simulink model "large_model"  (Simulink 8.0)
settings: Solver=ode45  StartTime=0.0  StopTime=10.0  RelTol=1e-3
(part 10 of 21)

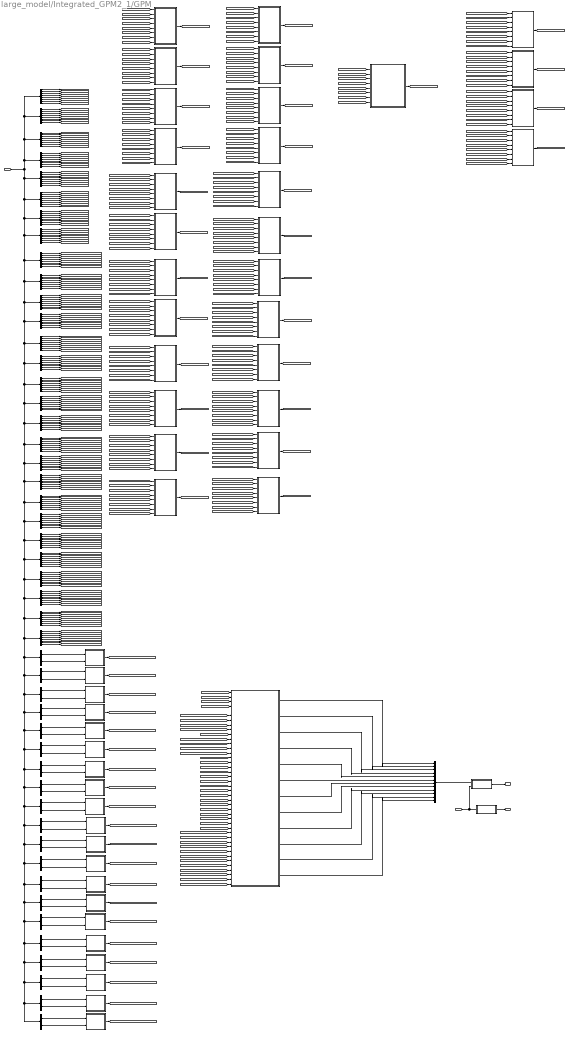
== system large_model/Integrated_GPM2_1/GPM ==
  note: Este componente foi colocado devido a uma limitação do EFTG. Na versão 1.2.1,
      | não é permitido fazer uma conexão direta entre a entrada e a saída.
  From_ES : Inport
  PWR_In : Inport  Port=2
  CAB1_FCC2 : Subsystem
  GPM Hardware : Subsystem
  Outputs : BusCreator  Inputs=12
  PWR : Subsystem
  SCP_REU_ROB_LVDT : Subsystem
  SCP_ail_REU_LIB_LVDT : Subsystem
  SCP_ail_REU_LIB_mon : Subsystem
  SCP_ail_REU_LOB_LVDT : Subsystem
  SCP_ail_REU_LOB_mon : Subsystem
  SCP_ail_REU_RIB_LVDT : Subsystem
  SCP_ail_REU_RIB_mon : Subsystem
  SCP_ail_REU_ROB_mon : Subsystem
  SCP_elev_REU_LIB_LVDT : Subsystem
  SCP_elev_REU_LIB_mon_dat : Subsystem
  SCP_elev_REU_LOB_LVDT : Subsystem
  SCP_elev_REU_LOB_mon : Subsystem
  SCP_elev_REU_RIB_LVDT_dat : Subsystem
  SCP_elev_REU_RIB_mon : Subsystem
  SCP_elev_REU_ROB_LVDT : Subsystem
  SCP_elev_REU_ROB_mon : Subsystem
  SCP_rud_REU1_LVDT : Subsystem
  SCP_rud_REU1_MON : Subsystem
  SCP_rud_REU2_LVDT : Subsystem
  SCP_rud_REU2_MON : Subsystem
  Self_checking_pair_ADS1A : Subsystem
  Self_checking_pair_ADS1B : Subsystem
  Self_checking_pair_ADS2A : Subsystem
  Self_checking_pair_ADS2B : Subsystem
  Self_checking_pair_ADS3A : Subsystem
  Self_checking_pair_ADS3B : Subsystem
  Self_checking_pair_ADS4A : Subsystem
  Self_checking_pair_ADS4B : Subsystem
  Self_checking_pair_PedalYaw1 : Subsystem
  Self_checking_pair_PedalYaw2 : Subsystem
  Self_checking_pair_PedalYaw3 : Subsystem
  Self_checking_pair_PedalYaw4 : Subsystem
  Self_checking_pair_SSC1_pitch1 : Subsystem
  Self_checking_pair_SSC1_pitch2 : Subsystem
  Self_checking_pair_SSC1_pitch3 : Subsystem
  Self_checking_pair_SSC1_pitch4 : Subsystem
  Self_checking_pair_SSC1_roll1 : Subsystem
  Self_checking_pair_SSC1_roll2 : Subsystem
  Self_checking_pair_SSC1_roll3 : Subsystem
  Self_checking_pair_SSC1_roll4 : Subsystem
  Self_checking_pair_SSC2_pitch1 : Subsystem
  Self_checking_pair_SSC2_pitch2 : Subsystem
  Self_checking_pair_SSC2_pitch3 : Subsystem
  Self_checking_pair_SSC2_pitch4 : Subsystem
  Self_checking_pair_SSC2_roll1 : Subsystem
  Self_checking_pair_SSC2_roll2 : Subsystem
  Self_checking_pair_SSC2_roll3 : Subsystem
  Self_checking_pair_SSC2_roll4 : Subsystem
  Voter_ADS : Subsystem
  ads_1A : BusSelector  Description=|  OutputSignals=switch1A.cab2_gpm_IO_chB.ads_1A,switch1A.cab1_gpm_IO_chB.ads_1A,switch1A.switch2A_to_switch.cab3_gpm_IO_chA.ads_1A,switch1A.switch2A_to_switch.cab4_gpm_IO_chA.ads_1A,switch1B.cab2_gpm_IO_chB.ads_1A,switch1B.cab1_gpm_IO_chB.ads_1A,switch1B.switch2B_to_switch.cab4_gpm_IO_chB.ads_1A,switch1B.switch2B_to_switch.cab3_gpm_IO_chB.ads_1A
      | When connected to a line which contains bus signals, this bus selector will select as its outputs all the signals which contain this bus selector name.
      | For example, if you name this bus selector as "sidestick_1_pitch_1", all signals containing this string will be selected as its outputs.
  ads_1B : BusSelector  Description=|  OutputSignals=switch1A.cab2_gpm_IO_chB.ads_1B,switch1A.cab1_gpm_IO_chB.ads_1B,switch1A.switch2A_to_switch.cab3_gpm_IO_chA.ads_1B,switch1A.switch2A_to_switch.cab4_gpm_IO_chA.ads_1B,switch1B.cab2_gpm_IO_chB.ads_1B,switch1B.cab1_gpm_IO_chB.ads_1B,switch1B.switch2B_to_switch.cab4_gpm_IO_chB.ads_1B,switch1B.switch2B_to_switch.cab3_gpm_IO_chB.ads_1B
      | When connected to a line which contains bus signals, this bus selector will select as its outputs all the signals which contain this bus selector name.
      | For example, if you name this bus selector as "sidestick_1_pitch_1", all signals containing this string will be selected as its outputs.
  ads_2A : BusSelector  Description=|  OutputSignals=switch1A.cab2_gpm_IO_chB.ads_2A,switch1A.cab1_gpm_IO_chB.ads_2A,switch1A.switch2A_to_switch.cab3_gpm_IO_chA.ads_2A,switch1A.switch2A_to_switch.cab4_gpm_IO_chA.ads_2A,switch1B.cab2_gpm_IO_chB.ads_2A,switch1B.cab1_gpm_IO_chB.ads_2A,switch1B.switch2B_to_switch.cab4_gpm_IO_chB.ads_2A,switch1B.switch2B_to_switch.cab3_gpm_IO_chB.ads_2A
      | When connected to a line which contains bus signals, this bus selector will select as its outputs all the signals which contain this bus selector name.
      | For example, if you name this bus selector as "sidestick_1_pitch_1", all signals containing this string will be selected as its outputs.
  ads_2B : BusSelector  Description=|  OutputSignals=switch1A.cab2_gpm_IO_chB.ads_2B,switch1A.cab1_gpm_IO_chB.ads_2B,switch1A.switch2A_to_switch.cab3_gpm_IO_chA.ads_2B,switch1A.switch2A_to_switch.cab4_gpm_IO_chA.ads_2B,switch1B.cab2_gpm_IO_chB.ads_2B,switch1B.cab1_gpm_IO_chB.ads_2B,switch1B.switch2B_to_switch.cab4_gpm_IO_chB.ads_2B,switch1B.switch2B_to_switch.cab3_gpm_IO_chB.ads_2B
      | When connected to a line which contains bus signals, this bus selector will select as its outputs all the signals which contain this bus selector name.
      | For example, if you name this bus selector as "sidestick_1_pitch_1", all signals containing this string will be selected as its outputs.
  ads_3A : BusSelector  Description=|  OutputSignals=switch1A.cab2_gpm_IO_chB.ads_3A,switch1A.cab1_gpm_IO_chB.ads_3A,switch1A.switch2A_to_switch.cab3_gpm_IO_chA.ads_3A,switch1A.switch2A_to_switch.cab4_gpm_IO_chA.ads_3A,switch1B.cab2_gpm_IO_chB.ads_3A,switch1B.cab1_gpm_IO_chB.ads_3A,switch1B.switch2B_to_switch.cab4_gpm_IO_chB.ads_3A,switch1B.switch2B_to_switch.cab3_gpm_IO_chB.ads_3A
      | When connected to a line which contains bus signals, this bus selector will select as its outputs all the signals which contain this bus selector name.
      | For example, if you name this bus selector as "sidestick_1_pitch_1", all signals containing this string will be selected as its outputs.
  ads_3B : BusSelector  Description=|  OutputSignals=switch1A.cab2_gpm_IO_chB.ads_3B,switch1A.cab1_gpm_IO_chB.ads_3B,switch1A.switch2A_to_switch.cab3_gpm_IO_chA.ads_3B,switch1A.switch2A_to_switch.cab4_gpm_IO_chA.ads_3B,switch1B.cab2_gpm_IO_chB.ads_3B,switch1B.cab1_gpm_IO_chB.ads_3B,switch1B.switch2B_to_switch.cab4_gpm_IO_chB.ads_3B,switch1B.switch2B_to_switch.cab3_gpm_IO_chB.ads_3B
      | When connected to a line which contains bus signals, this bus selector will select as its outputs all the signals which contain this bus selector name.
      | For example, if you name this bus selector as "sidestick_1_pitch_1", all signals containing this string will be selected as its outputs.
  ads_4A : BusSelector  Description=|  OutputSignals=switch1A.cab2_gpm_IO_chB.ads_4A,switch1A.cab1_gpm_IO_chB.ads_4A,switch1A.switch2A_to_switch.cab3_gpm_IO_chA.ads_4A,switch1A.switch2A_to_switch.cab4_gpm_IO_chA.ads_4A,switch1B.cab2_gpm_IO_chB.ads_4A,switch1B.cab1_gpm_IO_chB.ads_4A,switch1B.switch2B_to_switch.cab4_gpm_IO_chB.ads_4A,switch1B.switch2B_to_switch.cab3_gpm_IO_chB.ads_4A
      | When connected to a line which contains bus signals, this bus selector will select as its outputs all the signals which contain this bus selector name.
      | For example, if you name this bus selector as "sidestick_1_pitch_1", all signals containing this string will be selected as its outputs.
  ads_4B : BusSelector  Description=|  OutputSignals=switch1A.cab2_gpm_IO_chB.ads_4B,switch1A.cab1_gpm_IO_chB.ads_4B,switch1A.switch2A_to_switch.cab3_gpm_IO_chA.ads_4B,switch1A.switch2A_to_switch.cab4_gpm_IO_chA.ads_4B,switch1B.cab2_gpm_IO_chB.ads_4B,switch1B.cab1_gpm_IO_chB.ads_4B,switch1B.switch2B_to_switch.cab4_gpm_IO_chB.ads_4B,switch1B.switch2B_to_switch.cab3_gpm_IO_chB.ads_4B
      | When connected to a line which contains bus signals, this bus selector will select as its outputs all the signals which contain this bus selector name.
      | For example, if you name this bus selector as "sidestick_1_pitch_1", all signals containing this string will be selected as its outputs.
  ail_REU_LIB_LVDT_dat : BusSelector  OutputSignals=switch1A.switch2A_to_switch.REU_to_Switch2A.ail_REU_LIB_LVDT_dat,switch1B.switch2B_to_switch.REU_to_Switch2B.ail_REU_LIB_LVDT_dat
  ail_REU_LIB_mon_dat : BusSelector  OutputSignals=switch1A.switch2A_to_switch.REU_to_Switch2A.ail_REU_LIB_mon_dat,switch1B.switch2B_to_switch.REU_to_Switch2B.ail_REU_LIB_mon_dat
  ail_REU_LOB_LVDT_dat : BusSelector  OutputSignals=switch1A.REU_to_Switch1A.ail_REU_LOB_LVDT_dat,switch1B.REU_to_Switch1B.ail_REU_LOB_LVDT_dat
  ail_REU_LOB_mon_dat : BusSelector  OutputSignals=switch1A.REU_to_Switch1A.ail_REU_LOB_mon_dat,switch1B.REU_to_Switch1B.ail_REU_LOB_mon_dat
  ail_REU_RIB_LVDT_dat : BusSelector  OutputSignals=switch1A.switch2A_to_switch.REU_to_Switch2A.ail_REU_RIB_LVDT_dat,switch1B.switch2B_to_switch.REU_to_Switch2B.ail_REU_RIB_LVDT_dat
  ail_REU_RIB_mon_dat : BusSelector  OutputSignals=switch1A.switch2A_to_switch.REU_to_Switch2A.ail_REU_RIB_mon_dat,switch1B.switch2B_to_switch.REU_to_Switch2B.ail_REU_RIB_mon_dat
  ail_REU_ROB_LVDT_dat : BusSelector  OutputSignals=switch1A.REU_to_Switch1A.ail_REU_ROB_LVDT_dat,switch1B.REU_to_Switch1B.ail_REU_ROB_LVDT_dat
  ail_REU_ROB_mon_dat : BusSelector  OutputSignals=switch1A.REU_to_Switch1A.ail_REU_ROB_mon_dat,switch1B.REU_to_Switch1B.ail_REU_ROB_mon_dat
  elev_REU_LIB_LVDT_dat : BusSelector  OutputSignals=switch1A.switch2A_to_switch.REU_to_Switch2A.elev_REU_LIB_LVDT_dat,switch1B.switch2B_to_switch.REU_to_Switch2B.elev_REU_LIB_LVDT_dat
  elev_REU_LIB_mon_dat : BusSelector  OutputSignals=switch1A.switch2A_to_switch.REU_to_Switch2A.elev_REU_LIB_mon_dat,switch1B.switch2B_to_switch.REU_to_Switch2B.elev_REU_LIB_mon_dat
  elev_REU_LOB_LVDT_dat : BusSelector  OutputSignals=switch1A.REU_to_Switch1A.elev_REU_LOB_LVDT_dat,switch1B.REU_to_Switch1B.elev_REU_LOB_LVDT_dat
  elev_REU_LOB_mon_dat : BusSelector  OutputSignals=switch1A.REU_to_Switch1A.elev_REU_LOB_mon_dat,switch1B.REU_to_Switch1B.elev_REU_LOB_mon_dat
  elev_REU_RIB_LVDT_dat : BusSelector  OutputSignals=switch1A.switch2A_to_switch.REU_to_Switch2A.elev_REU_RIB_LVDT_dat,switch1B.switch2B_to_switch.REU_to_Switch2B.elev_REU_RIB_LVDT_dat
  elev_REU_RIB_mon_dat : BusSelector  OutputSignals=switch1A.switch2A_to_switch.REU_to_Switch2A.elev_REU_RIB_mon_dat,switch1B.switch2B_to_switch.REU_to_Switch2B.elev_REU_RIB_mon_dat
  elev_REU_ROB_LVDT_dat : BusSelector  OutputSignals=switch1A.REU_to_Switch1A.elev_REU_ROB_LVDT_dat,switch1B.REU_to_Switch1B.elev_REU_ROB_LVDT_dat
  elev_REU_ROB_mon_dat : BusSelector  OutputSignals=switch1A.REU_to_Switch1A.elev_REU_ROB_mon_dat,switch1B.REU_to_Switch1B.elev_REU_ROB_mon_dat
  pedal_yaw_1 : BusSelector  Description=|  OutputSignals=switch1A.cab2_gpm_IO_chB.rdc_4002_1.pedal_yaw_1,switch1A.cab1_gpm_IO_chB.rdc_4002_1.pedal_yaw_1,switch1A.switch2A_to_switch.cab3_gpm_IO_chA.rdc_4002_1.pedal_yaw_1,switch1A.switch2A_to_switch.cab4_gpm_IO_chA.rdc_4002_1.pedal_yaw_1,switch1B.cab2_gpm_IO_chB.rdc_4002_1.pedal_yaw_1,switch1B.cab1_gpm_IO_chB.rdc_4002_1.pedal_yaw_1,switch1B.switch2B_to_switch.cab4_gpm_IO_chB.rdc_4002_1.pedal_yaw_1,switch1B.switch2B_to_switch.cab3_gpm_IO_chB.rdc_4002_1.pedal_yaw_1
      | When connected to a line which contains bus signals, this bus selector will select as its outputs all the signals which contain this bus selector name.
      | For example, if you name this bus selector as "sidestick_1_pitch_1", all signals containing this string will be selected as its outputs.
  pedal_yaw_2 : BusSelector  Description=|  OutputSignals=switch1A.cab2_gpm_IO_chB.rdc_4002_2.pedal_yaw_2,switch1A.cab1_gpm_IO_chB.rdc_4002_2.pedal_yaw_2,switch1A.switch2A_to_switch.cab3_gpm_IO_chA.rdc_4002_2.pedal_yaw_2,switch1A.switch2A_to_switch.cab4_gpm_IO_chA.rdc_4002_2.pedal_yaw_2,switch1B.cab2_gpm_IO_chB.rdc_4002_2.pedal_yaw_2,switch1B.cab1_gpm_IO_chB.rdc_4002_2.pedal_yaw_2,switch1B.switch2B_to_switch.cab4_gpm_IO_chB.rdc_4002_2.pedal_yaw_2,switch1B.switch2B_to_switch.cab3_gpm_IO_chB.rdc_4002_2.pedal_yaw_2
      | When connected to a line which contains bus signals, this bus selector will select as its outputs all the signals which contain this bus selector name.
      | For example, if you name this bus selector as "sidestick_1_pitch_1", all signals containing this string will be selected as its outputs.
  pedal_yaw_3 : BusSelector  Description=|  OutputSignals=switch1A.cab2_gpm_IO_chB.rdc_4002_3.pedal_yaw_3,switch1A.cab1_gpm_IO_chB.rdc_4002_3.pedal_yaw_3,switch1A.switch2A_to_switch.cab3_gpm_IO_chA.rdc_4002_3.pedal_yaw_3,switch1A.switch2A_to_switch.cab4_gpm_IO_chA.rdc_4002_3.pedal_yaw_3,switch1B.cab2_gpm_IO_chB.rdc_4002_3.pedal_yaw_3,switch1B.cab1_gpm_IO_chB.rdc_4002_3.pedal_yaw_3,switch1B.switch2B_to_switch.cab4_gpm_IO_chB.rdc_4002_3.pedal_yaw_3,switch1B.switch2B_to_switch.cab3_gpm_IO_chB.rdc_4002_3.pedal_yaw_3
      | When connected to a line which contains bus signals, this bus selector will select as its outputs all the signals which contain this bus selector name.
      | For example, if you name this bus selector as "sidestick_1_pitch_1", all signals containing this string will be selected as its outputs.
  pedal_yaw_4 : BusSelector  Description=|  OutputSignals=switch1A.cab2_gpm_IO_chB.rdc_4002_4.pedal_yaw_4,switch1A.cab1_gpm_IO_chB.rdc_4002_4.pedal_yaw_4,switch1A.switch2A_to_switch.cab3_gpm_IO_chA.rdc_4002_4.pedal_yaw_4,switch1A.switch2A_to_switch.cab4_gpm_IO_chA.rdc_4002_4.pedal_yaw_4,switch1B.cab2_gpm_IO_chB.rdc_4002_4.pedal_yaw_4,switch1B.cab1_gpm_IO_chB.rdc_4002_4.pedal_yaw_4,switch1B.switch2B_to_switch.cab4_gpm_IO_chB.rdc_4002_4.pedal_yaw_4,switch1B.switch2B_to_switch.cab3_gpm_IO_chB.rdc_4002_4.pedal_yaw_4
      | When connected to a line which contains bus signals, this bus selector will select as its outputs all the signals which contain this bus selector name.
      | For example, if you name this bus selector as "sidestick_1_pitch_1", all signals containing this string will be selected as its outputs.
  rud_REU1_LVDT_dat : BusSelector  OutputSignals=switch1A.switch2A_to_switch.REU_to_Switch2A.rud_REU1_LVDT_dat,switch1B.switch2B_to_switch.REU_to_Switch2B.rud_REU1_LVDT_dat
  rud_REU1_MON_dat : BusSelector  OutputSignals=switch1A.switch2A_to_switch.REU_to_Switch2A.rud_REU1_MON_dat,switch1B.switch2B_to_switch.REU_to_Switch2B.rud_REU1_MON_dat
  rud_REU2_LVDT_dat : BusSelector  OutputSignals=switch1A.REU_to_Switch1A.rud_REU2_LVDT_dat,switch1B.REU_to_Switch1B.rud_REU2_LVDT_dat
  rud_REU2_MON_dat : BusSelector  OutputSignals=switch1A.REU_to_Switch1A.rud_REU2_MON_dat,switch1B.REU_to_Switch1B.rud_REU2_MON_dat
  sidestick1_pitch_1 : BusSelector  OutputSignals=switch1A.cab2_gpm_IO_chB.rdc_4002_1.sidesticks.sidestick1_pitch_1,switch1A.cab1_gpm_IO_chB.rdc_4002_1.sidesticks.sidestick1_pitch_1,switch1A.switch2A_to_switch.cab3_gpm_IO_chA.rdc_4002_1.sidesticks.sidestick1_pitch_1,switch1A.switch2A_to_switch.cab4_gpm_IO_chA.rdc_4002_1.sidesticks.sidestick1_pitch_1,switch1B.cab2_gpm_IO_chB.rdc_4002_1.sidesticks.sidestick1_pitch_1,switch1B.cab1_gpm_IO_chB.rdc_4002_1.sidesticks.sidestick1_pitch_1,switch1B.switch2B_to_switch.cab4_gpm_IO_chB.rdc_4002_1.sidesticks.sidestick1_pitch_1,switch1B.switch2B_to_switch.cab3_gpm_IO_chB.rdc_4002_1.sidesticks.sidestick1_pitch_1
  sidestick1_pitch_2 : BusSelector  OutputSignals=switch1A.cab2_gpm_IO_chB.rdc_4002_2.sidesticks.sidestick1_pitch_2,switch1A.cab1_gpm_IO_chB.rdc_4002_2.sidesticks.sidestick1_pitch_2,switch1A.switch2A_to_switch.cab3_gpm_IO_chA.rdc_4002_2.sidesticks.sidestick1_pitch_2,switch1A.switch2A_to_switch.cab4_gpm_IO_chA.rdc_4002_2.sidesticks.sidestick1_pitch_2,switch1B.cab2_gpm_IO_chB.rdc_4002_2.sidesticks.sidestick1_pitch_2,switch1B.cab1_gpm_IO_chB.rdc_4002_2.sidesticks.sidestick1_pitch_2,switch1B.switch2B_to_switch.cab4_gpm_IO_chB.rdc_4002_2.sidesticks.sidestick1_pitch_2,switch1B.switch2B_to_switch.cab3_gpm_IO_chB.rdc_4002_2.sidesticks.sidestick1_pitch_2
  sidestick1_pitch_3 : BusSelector  OutputSignals=switch1A.cab2_gpm_IO_chB.rdc_4002_3.sidesticks.sidestick1_pitch_3,switch1A.cab1_gpm_IO_chB.rdc_4002_3.sidesticks.sidestick1_pitch_3,switch1A.switch2A_to_switch.cab3_gpm_IO_chA.rdc_4002_3.sidesticks.sidestick1_pitch_3,switch1A.switch2A_to_switch.cab4_gpm_IO_chA.rdc_4002_3.sidesticks.sidestick1_pitch_3,switch1B.cab2_gpm_IO_chB.rdc_4002_3.sidesticks.sidestick1_pitch_3,switch1B.cab1_gpm_IO_chB.rdc_4002_3.sidesticks.sidestick1_pitch_3,switch1B.switch2B_to_switch.cab4_gpm_IO_chB.rdc_4002_3.sidesticks.sidestick1_pitch_3,switch1B.switch2B_to_switch.cab3_gpm_IO_chB.rdc_4002_3.sidesticks.sidestick1_pitch_3
  sidestick1_pitch_4 : BusSelector  OutputSignals=switch1A.cab2_gpm_IO_chB.rdc_4002_4.sidesticks.sidestick1_pitch_4,switch1A.cab1_gpm_IO_chB.rdc_4002_4.sidesticks.sidestick1_pitch_4,switch1A.switch2A_to_switch.cab3_gpm_IO_chA.rdc_4002_4.sidesticks.sidestick1_pitch_4,switch1A.switch2A_to_switch.cab4_gpm_IO_chA.rdc_4002_4.sidesticks.sidestick1_pitch_4,switch1B.cab2_gpm_IO_chB.rdc_4002_4.sidesticks.sidestick1_pitch_4,switch1B.cab1_gpm_IO_chB.rdc_4002_4.sidesticks.sidestick1_pitch_4,switch1B.switch2B_to_switch.cab4_gpm_IO_chB.rdc_4002_4.sidesticks.sidestick1_pitch_4,switch1B.switch2B_to_switch.cab3_gpm_IO_chB.rdc_4002_4.sidesticks.sidestick1_pitch_4
  sidestick1_roll_1 : BusSelector  OutputSignals=switch1A.cab2_gpm_IO_chB.rdc_4002_1.sidesticks.sidestick1_roll_1,switch1A.cab1_gpm_IO_chB.rdc_4002_1.sidesticks.sidestick1_roll_1,switch1A.switch2A_to_switch.cab3_gpm_IO_chA.rdc_4002_1.sidesticks.sidestick1_roll_1,switch1A.switch2A_to_switch.cab4_gpm_IO_chA.rdc_4002_1.sidesticks.sidestick1_roll_1,switch1B.cab2_gpm_IO_chB.rdc_4002_1.sidesticks.sidestick1_roll_1,switch1B.cab1_gpm_IO_chB.rdc_4002_1.sidesticks.sidestick1_roll_1,switch1B.switch2B_to_switch.cab4_gpm_IO_chB.rdc_4002_1.sidesticks.sidestick1_roll_1,switch1B.switch2B_to_switch.cab3_gpm_IO_chB.rdc_4002_1.sidesticks.sidestick1_roll_1
  sidestick1_roll_2 : BusSelector  OutputSignals=switch1A.cab2_gpm_IO_chB.rdc_4002_2.sidesticks.sidestick1_roll_2,switch1A.cab1_gpm_IO_chB.rdc_4002_2.sidesticks.sidestick1_roll_2,switch1A.switch2A_to_switch.cab3_gpm_IO_chA.rdc_4002_2.sidesticks.sidestick1_roll_2,switch1A.switch2A_to_switch.cab4_gpm_IO_chA.rdc_4002_2.sidesticks.sidestick1_roll_2,switch1B.cab2_gpm_IO_chB.rdc_4002_2.sidesticks.sidestick1_roll_2,switch1B.cab1_gpm_IO_chB.rdc_4002_2.sidesticks.sidestick1_roll_2,switch1B.switch2B_to_switch.cab4_gpm_IO_chB.rdc_4002_2.sidesticks.sidestick1_roll_2,switch1B.switch2B_to_switch.cab3_gpm_IO_chB.rdc_4002_2.sidesticks.sidestick1_roll_2
  sidestick1_roll_3 : BusSelector  OutputSignals=switch1A.cab2_gpm_IO_chB.rdc_4002_3.sidesticks.sidestick1_roll_3,switch1A.cab1_gpm_IO_chB.rdc_4002_3.sidesticks.sidestick1_roll_3,switch1A.switch2A_to_switch.cab3_gpm_IO_chA.rdc_4002_3.sidesticks.sidestick1_roll_3,switch1A.switch2A_to_switch.cab4_gpm_IO_chA.rdc_4002_3.sidesticks.sidestick1_roll_3,switch1B.cab2_gpm_IO_chB.rdc_4002_3.sidesticks.sidestick1_roll_3,switch1B.cab1_gpm_IO_chB.rdc_4002_3.sidesticks.sidestick1_roll_3,switch1B.switch2B_to_switch.cab4_gpm_IO_chB.rdc_4002_3.sidesticks.sidestick1_roll_3,switch1B.switch2B_to_switch.cab3_gpm_IO_chB.rdc_4002_3.sidesticks.sidestick1_roll_3
  sidestick1_roll_4 : BusSelector  OutputSignals=switch1A.cab2_gpm_IO_chB.rdc_4002_4.sidesticks.sidestick1_roll_4,switch1A.cab1_gpm_IO_chB.rdc_4002_4.sidesticks.sidestick1_roll_4,switch1A.switch2A_to_switch.cab3_gpm_IO_chA.rdc_4002_4.sidesticks.sidestick1_roll_4,switch1A.switch2A_to_switch.cab4_gpm_IO_chA.rdc_4002_4.sidesticks.sidestick1_roll_4,switch1B.cab2_gpm_IO_chB.rdc_4002_4.sidesticks.sidestick1_roll_4,switch1B.cab1_gpm_IO_chB.rdc_4002_4.sidesticks.sidestick1_roll_4,switch1B.switch2B_to_switch.cab4_gpm_IO_chB.rdc_4002_4.sidesticks.sidestick1_roll_4,switch1B.switch2B_to_switch.cab3_gpm_IO_chB.rdc_4002_4.sidesticks.sidestick1_roll_4
  sidestick2_pitch_1 : BusSelector  OutputSignals=switch1A.cab2_gpm_IO_chB.rdc_4002_1.sidesticks.sidestick2_pitch_1,switch1A.cab1_gpm_IO_chB.rdc_4002_1.sidesticks.sidestick2_pitch_1,switch1A.switch2A_to_switch.cab3_gpm_IO_chA.rdc_4002_1.sidesticks.sidestick2_pitch_1,switch1A.switch2A_to_switch.cab4_gpm_IO_chA.rdc_4002_1.sidesticks.sidestick2_pitch_1,switch1B.cab2_gpm_IO_chB.rdc_4002_1.sidesticks.sidestick2_pitch_1,switch1B.cab1_gpm_IO_chB.rdc_4002_1.sidesticks.sidestick2_pitch_1,switch1B.switch2B_to_switch.cab4_gpm_IO_chB.rdc_4002_1.sidesticks.sidestick2_pitch_1,switch1B.switch2B_to_switch.cab3_gpm_IO_chB.rdc_4002_1.sidesticks.sidestick2_pitch_1
  sidestick2_pitch_2 : BusSelector  OutputSignals=switch1A.cab2_gpm_IO_chB.rdc_4002_2.sidesticks.sidestick2_pitch_2,switch1A.cab1_gpm_IO_chB.rdc_4002_2.sidesticks.sidestick2_pitch_2,switch1A.switch2A_to_switch.cab3_gpm_IO_chA.rdc_4002_2.sidesticks.sidestick2_pitch_2,switch1A.switch2A_to_switch.cab4_gpm_IO_chA.rdc_4002_2.sidesticks.sidestick2_pitch_2,switch1B.cab2_gpm_IO_chB.rdc_4002_2.sidesticks.sidestick2_pitch_2,switch1B.cab1_gpm_IO_chB.rdc_4002_2.sidesticks.sidestick2_pitch_2,switch1B.switch2B_to_switch.cab4_gpm_IO_chB.rdc_4002_2.sidesticks.sidestick2_pitch_2,switch1B.switch2B_to_switch.cab3_gpm_IO_chB.rdc_4002_2.sidesticks.sidestick2_pitch_2
  sidestick2_pitch_3 : BusSelector  OutputSignals=switch1A.cab2_gpm_IO_chB.rdc_4002_3.sidesticks.sidestick2_pitch_3,switch1A.cab1_gpm_IO_chB.rdc_4002_3.sidesticks.sidestick2_pitch_3,switch1A.switch2A_to_switch.cab3_gpm_IO_chA.rdc_4002_3.sidesticks.sidestick2_pitch_3,switch1A.switch2A_to_switch.cab4_gpm_IO_chA.rdc_4002_3.sidesticks.sidestick2_pitch_3,switch1B.cab2_gpm_IO_chB.rdc_4002_3.sidesticks.sidestick2_pitch_3,switch1B.cab1_gpm_IO_chB.rdc_4002_3.sidesticks.sidestick2_pitch_3,switch1B.switch2B_to_switch.cab4_gpm_IO_chB.rdc_4002_3.sidesticks.sidestick2_pitch_3,switch1B.switch2B_to_switch.cab3_gpm_IO_chB.rdc_4002_3.sidesticks.sidestick2_pitch_3
  sidestick2_pitch_4 : BusSelector  OutputSignals=switch1A.cab2_gpm_IO_chB.rdc_4002_4.sidesticks.sidestick2_pitch_4,switch1A.cab1_gpm_IO_chB.rdc_4002_4.sidesticks.sidestick2_pitch_4,switch1A.switch2A_to_switch.cab3_gpm_IO_chA.rdc_4002_4.sidesticks.sidestick2_pitch_4,switch1A.switch2A_to_switch.cab4_gpm_IO_chA.rdc_4002_4.sidesticks.sidestick2_pitch_4,switch1B.cab2_gpm_IO_chB.rdc_4002_4.sidesticks.sidestick2_pitch_4,switch1B.cab1_gpm_IO_chB.rdc_4002_4.sidesticks.sidestick2_pitch_4,switch1B.switch2B_to_switch.cab4_gpm_IO_chB.rdc_4002_4.sidesticks.sidestick2_pitch_4,switch1B.switch2B_to_switch.cab3_gpm_IO_chB.rdc_4002_4.sidesticks.sidestick2_pitch_4
  sidestick2_roll_1 : BusSelector  OutputSignals=switch1A.cab2_gpm_IO_chB.rdc_4002_1.sidesticks.sidestick2_roll_1,switch1A.cab1_gpm_IO_chB.rdc_4002_1.sidesticks.sidestick2_roll_1,switch1A.switch2A_to_switch.cab3_gpm_IO_chA.rdc_4002_1.sidesticks.sidestick2_roll_1,switch1A.switch2A_to_switch.cab4_gpm_IO_chA.rdc_4002_1.sidesticks.sidestick2_roll_1,switch1B.cab2_gpm_IO_chB.rdc_4002_1.sidesticks.sidestick2_roll_1,switch1B.cab1_gpm_IO_chB.rdc_4002_1.sidesticks.sidestick2_roll_1,switch1B.switch2B_to_switch.cab4_gpm_IO_chB.rdc_4002_1.sidesticks.sidestick2_roll_1,switch1B.switch2B_to_switch.cab3_gpm_IO_chB.rdc_4002_1.sidesticks.sidestick2_roll_1
  sidestick2_roll_2 : BusSelector  OutputSignals=switch1A.cab2_gpm_IO_chB.rdc_4002_2.sidesticks.sidestick2_roll_2,switch1A.cab1_gpm_IO_chB.rdc_4002_2.sidesticks.sidestick2_roll_2,switch1A.switch2A_to_switch.cab3_gpm_IO_chA.rdc_4002_2.sidesticks.sidestick2_roll_2,switch1A.switch2A_to_switch.cab4_gpm_IO_chA.rdc_4002_2.sidesticks.sidestick2_roll_2,switch1B.cab2_gpm_IO_chB.rdc_4002_2.sidesticks.sidestick2_roll_2,switch1B.cab1_gpm_IO_chB.rdc_4002_2.sidesticks.sidestick2_roll_2,switch1B.switch2B_to_switch.cab4_gpm_IO_chB.rdc_4002_2.sidesticks.sidestick2_roll_2,switch1B.switch2B_to_switch.cab3_gpm_IO_chB.rdc_4002_2.sidesticks.sidestick2_roll_2
  sidestick2_roll_3 : BusSelector  OutputSignals=switch1A.cab2_gpm_IO_chB.rdc_4002_3.sidesticks.sidestick2_roll_3,switch1A.cab1_gpm_IO_chB.rdc_4002_3.sidesticks.sidestick2_roll_3,switch1A.switch2A_to_switch.cab3_gpm_IO_chA.rdc_4002_3.sidesticks.sidestick2_roll_3,switch1A.switch2A_to_switch.cab4_gpm_IO_chA.rdc_4002_3.sidesticks.sidestick2_roll_3,switch1B.cab2_gpm_IO_chB.rdc_4002_3.sidesticks.sidestick2_roll_3,switch1B.cab1_gpm_IO_chB.rdc_4002_3.sidesticks.sidestick2_roll_3,switch1B.switch2B_to_switch.cab4_gpm_IO_chB.rdc_4002_3.sidesticks.sidestick2_roll_3,switch1B.switch2B_to_switch.cab3_gpm_IO_chB.rdc_4002_3.sidesticks.sidestick2_roll_3
  sidestick2_roll_4 : BusSelector  OutputSignals=switch1A.cab2_gpm_IO_chB.rdc_4002_4.sidesticks.sidestick2_roll_4,switch1A.cab1_gpm_IO_chB.rdc_4002_4.sidesticks.sidestick2_roll_4,switch1A.switch2A_to_switch.cab3_gpm_IO_chA.rdc_4002_4.sidesticks.sidestick2_roll_4,switch1A.switch2A_to_switch.cab4_gpm_IO_chA.rdc_4002_4.sidesticks.sidestick2_roll_4,switch1B.cab2_gpm_IO_chB.rdc_4002_4.sidesticks.sidestick2_roll_4,switch1B.cab1_gpm_IO_chB.rdc_4002_4.sidesticks.sidestick2_roll_4,switch1B.switch2B_to_switch.cab4_gpm_IO_chB.rdc_4002_4.sidesticks.sidestick2_roll_4,switch1B.switch2B_to_switch.cab3_gpm_IO_chB.rdc_4002_4.sidesticks.sidestick2_roll_4
  To_ES : Outport
  PWR_Out : Outport  Port=2
  wires:
  rud_REU2_MON_dat/2 -> SCP_rud_REU2_MON/2(In2)  "<rud_REU2_MON_dat>"
  rud_REU2_MON_dat/1 -> SCP_rud_REU2_MON/1(In1)  "<rud_REU2_MON_dat>"
  rud_REU2_LVDT_dat/2 -> SCP_rud_REU2_LVDT/2(In2)  "<rud_REU2_LVDT_dat>"
  rud_REU2_LVDT_dat/1 -> SCP_rud_REU2_LVDT/1(In1)  "<rud_REU2_LVDT_dat>"
  rud_REU1_MON_dat/2 -> SCP_rud_REU1_MON/2(In2)  "<rud_REU1_MON_dat>"
  rud_REU1_MON_dat/1 -> SCP_rud_REU1_MON/1(In1)  "<rud_REU1_MON_dat>"
  rud_REU1_LVDT_dat/2 -> SCP_rud_REU1_LVDT/2(In2)  "<rud_REU1_LVDT_dat>"
  rud_REU1_LVDT_dat/1 -> SCP_rud_REU1_LVDT/1(In1)  "<rud_REU1_LVDT_dat>"
  elev_REU_LOB_mon_dat/2 -> SCP_elev_REU_LOB_mon/2(In2)  "<elev_REU_LOB_mon_dat>"
  elev_REU_LOB_mon_dat/1 -> SCP_elev_REU_LOB_mon/1(In1)  "<elev_REU_LOB_mon_dat>"
  elev_REU_LOB_LVDT_dat/2 -> SCP_elev_REU_LOB_LVDT/2(In2)  "<elev_REU_LOB_LVDT_dat>"
  elev_REU_LOB_LVDT_dat/1 -> SCP_elev_REU_LOB_LVDT/1(In1)  "<elev_REU_LOB_LVDT_dat>"
  elev_REU_LIB_mon_dat/2 -> SCP_elev_REU_LIB_mon_dat/2(In2)  "<elev_REU_LIB_mon_dat>"
  elev_REU_LIB_mon_dat/1 -> SCP_elev_REU_LIB_mon_dat/1(In1)  "<elev_REU_LIB_mon_dat>"
  elev_REU_LIB_LVDT_dat/2 -> SCP_elev_REU_LIB_LVDT/2(In2)  "<elev_REU_LIB_LVDT_dat>"
  elev_REU_LIB_LVDT_dat/1 -> SCP_elev_REU_LIB_LVDT/1(In1)  "<elev_REU_LIB_LVDT_dat>"
  ail_REU_ROB_mon_dat/2 -> SCP_ail_REU_ROB_mon/2(In2)  "<ail_REU_ROB_mon_dat>"
  ail_REU_ROB_mon_dat/1 -> SCP_ail_REU_ROB_mon/1(In1)  "<ail_REU_ROB_mon_dat>"
  ail_REU_ROB_LVDT_dat/2 -> SCP_REU_ROB_LVDT/2(In2)  "<ail_REU_ROB_LVDT_dat>"
  ail_REU_ROB_LVDT_dat/1 -> SCP_REU_ROB_LVDT/1(In1)  "<ail_REU_ROB_LVDT_dat>"
  ail_REU_RIB_mon_dat/2 -> SCP_ail_REU_RIB_mon/2(In2)  "<ail_REU_RIB_mon_dat>"
  ail_REU_RIB_mon_dat/1 -> SCP_ail_REU_RIB_mon/1(In1)  "<ail_REU_RIB_mon_dat>"
  ail_REU_RIB_LVDT_dat/2 -> SCP_ail_REU_RIB_LVDT/2(In2)  "<ail_REU_RIB_LVDT_dat>"
  ail_REU_RIB_LVDT_dat/1 -> SCP_ail_REU_RIB_LVDT/1(In1)  "<ail_REU_RIB_LVDT_dat>"
  ail_REU_LOB_mon_dat/2 -> SCP_ail_REU_LOB_mon/2(In2)  "<ail_REU_LOB_mon_dat>"
  ail_REU_LOB_mon_dat/1 -> SCP_ail_REU_LOB_mon/1(In1)  "<ail_REU_LOB_mon_dat>"
  ail_REU_LOB_LVDT_dat/2 -> SCP_ail_REU_LOB_LVDT/2(In2)  "<ail_REU_LOB_LVDT_dat>"
  ail_REU_LOB_LVDT_dat/1 -> SCP_ail_REU_LOB_LVDT/1(In1)  "<ail_REU_LOB_LVDT_dat>"
  ail_REU_LIB_mon_dat/2 -> SCP_ail_REU_LIB_mon/2(In2)  "<ail_REU_LIB_mon_dat>"
  ail_REU_LIB_mon_dat/1 -> SCP_ail_REU_LIB_mon/1(In1)  "<ail_REU_LIB_mon_dat>"
  ail_REU_LIB_LVDT_dat/2 -> SCP_ail_REU_LIB_LVDT/2(In2)  "<ail_REU_LIB_LVDT_dat>"
  ail_REU_LIB_LVDT_dat/1 -> SCP_ail_REU_LIB_LVDT/1(In1)  "<ail_REU_LIB_LVDT_dat>"
  [pedal_yaw_1_1_pedal_yaw_1] -> Self_checking_pair_PedalYaw1/1(In1)
  pedal_yaw_1/1 -> [pedal_yaw_1_1_pedal_yaw_1]  "<pedal_yaw_1>"
  [pedal_yaw_1_2_pedal_yaw_1] -> Self_checking_pair_PedalYaw1/2(In2)
  pedal_yaw_1/2 -> [pedal_yaw_1_2_pedal_yaw_1]  "<pedal_yaw_1>"
  [pedal_yaw_1_3_pedal_yaw_1] -> Self_checking_pair_PedalYaw1/3(In3)
  pedal_yaw_1/3 -> [pedal_yaw_1_3_pedal_yaw_1]  "<pedal_yaw_1>"
  [pedal_yaw_1_4_pedal_yaw_1] -> Self_checking_pair_PedalYaw1/4(In4)
  pedal_yaw_1/4 -> [pedal_yaw_1_4_pedal_yaw_1]  "<pedal_yaw_1>"
  [pedal_yaw_1_5_pedal_yaw_1] -> Self_checking_pair_PedalYaw1/5(In5)
  pedal_yaw_1/5 -> [pedal_yaw_1_5_pedal_yaw_1]  "<pedal_yaw_1>"
  [pedal_yaw_1_6_pedal_yaw_1] -> Self_checking_pair_PedalYaw1/6(In6)
  pedal_yaw_1/6 -> [pedal_yaw_1_6_pedal_yaw_1]  "<pedal_yaw_1>"
  [pedal_yaw_1_7_pedal_yaw_1] -> Self_checking_pair_PedalYaw1/7(In7)
  pedal_yaw_1/7 -> [pedal_yaw_1_7_pedal_yaw_1]  "<pedal_yaw_1>"
  [pedal_yaw_1_8_pedal_yaw_1] -> Self_checking_pair_PedalYaw1/8(In8)
  pedal_yaw_1/8 -> [pedal_yaw_1_8_pedal_yaw_1]  "<pedal_yaw_1>"
  [pedal_yaw_2_1_pedal_yaw_2] -> Self_checking_pair_PedalYaw2/1(In1)
  pedal_yaw_2/1 -> [pedal_yaw_2_1_pedal_yaw_2]  "<pedal_yaw_2>"
  [pedal_yaw_2_2_pedal_yaw_2] -> Self_checking_pair_PedalYaw2/2(In2)
  pedal_yaw_2/2 -> [pedal_yaw_2_2_pedal_yaw_2]  "<pedal_yaw_2>"
  [pedal_yaw_2_3_pedal_yaw_2] -> Self_checking_pair_PedalYaw2/3(In3)
  pedal_yaw_2/3 -> [pedal_yaw_2_3_pedal_yaw_2]  "<pedal_yaw_2>"
  [pedal_yaw_2_4_pedal_yaw_2] -> Self_checking_pair_PedalYaw2/4(In4)
  pedal_yaw_2/4 -> [pedal_yaw_2_4_pedal_yaw_2]  "<pedal_yaw_2>"
  [pedal_yaw_2_5_pedal_yaw_2] -> Self_checking_pair_PedalYaw2/5(In5)
  pedal_yaw_2/5 -> [pedal_yaw_2_5_pedal_yaw_2]  "<pedal_yaw_2>"
  [pedal_yaw_2_6_pedal_yaw_2] -> Self_checking_pair_PedalYaw2/6(In6)
  pedal_yaw_2/6 -> [pedal_yaw_2_6_pedal_yaw_2]  "<pedal_yaw_2>"
  [pedal_yaw_2_7_pedal_yaw_2] -> Self_checking_pair_PedalYaw2/7(In7)
  pedal_yaw_2/7 -> [pedal_yaw_2_7_pedal_yaw_2]  "<pedal_yaw_2>"
  [pedal_yaw_2_8_pedal_yaw_2] -> Self_checking_pair_PedalYaw2/8(In8)
  pedal_yaw_2/8 -> [pedal_yaw_2_8_pedal_yaw_2]  "<pedal_yaw_2>"
  [pedal_yaw_3_1_pedal_yaw_3] -> Self_checking_pair_PedalYaw3/1(In1)
  pedal_yaw_3/1 -> [pedal_yaw_3_1_pedal_yaw_3]  "<pedal_yaw_3>"
  [pedal_yaw_3_2_pedal_yaw_3] -> Self_checking_pair_PedalYaw3/2(In2)
  pedal_yaw_3/2 -> [pedal_yaw_3_2_pedal_yaw_3]  "<pedal_yaw_3>"
  [pedal_yaw_3_3_pedal_yaw_3] -> Self_checking_pair_PedalYaw3/3(In3)
  pedal_yaw_3/3 -> [pedal_yaw_3_3_pedal_yaw_3]  "<pedal_yaw_3>"
  [pedal_yaw_3_4_pedal_yaw_3] -> Self_checking_pair_PedalYaw3/4(In4)
  pedal_yaw_3/4 -> [pedal_yaw_3_4_pedal_yaw_3]  "<pedal_yaw_3>"
  [pedal_yaw_3_5_pedal_yaw_3] -> Self_checking_pair_PedalYaw3/5(In5)
  pedal_yaw_3/5 -> [pedal_yaw_3_5_pedal_yaw_3]  "<pedal_yaw_3>"
  [pedal_yaw_3_6_pedal_yaw_3] -> Self_checking_pair_PedalYaw3/6(In6)
  pedal_yaw_3/6 -> [pedal_yaw_3_6_pedal_yaw_3]  "<pedal_yaw_3>"
  [pedal_yaw_3_7_pedal_yaw_3] -> Self_checking_pair_PedalYaw3/7(In7)
  pedal_yaw_3/7 -> [pedal_yaw_3_7_pedal_yaw_3]  "<pedal_yaw_3>"
  [pedal_yaw_3_8_pedal_yaw_3] -> Self_checking_pair_PedalYaw3/8(In8)
  pedal_yaw_3/8 -> [pedal_yaw_3_8_pedal_yaw_3]  "<pedal_yaw_3>"
  [pedal_yaw_4_1_pedal_yaw_4] -> Self_checking_pair_PedalYaw4/1(In1)
  pedal_yaw_4/1 -> [pedal_yaw_4_1_pedal_yaw_4]  "<pedal_yaw_4>"
  [pedal_yaw_4_2_pedal_yaw_4] -> Self_checking_pair_PedalYaw4/2(In2)
  pedal_yaw_4/2 -> [pedal_yaw_4_2_pedal_yaw_4]  "<pedal_yaw_4>"
  [pedal_yaw_4_3_pedal_yaw_4] -> Self_checking_pair_PedalYaw4/3(In3)
  pedal_yaw_4/3 -> [pedal_yaw_4_3_pedal_yaw_4]  "<pedal_yaw_4>"
  [pedal_yaw_4_4_pedal_yaw_4] -> Self_checking_pair_PedalYaw4/4(In4)
  pedal_yaw_4/4 -> [pedal_yaw_4_4_pedal_yaw_4]  "<pedal_yaw_4>"
  [pedal_yaw_4_5_pedal_yaw_4] -> Self_checking_pair_PedalYaw4/5(In5)
  pedal_yaw_4/5 -> [pedal_yaw_4_5_pedal_yaw_4]  "<pedal_yaw_4>"
  [pedal_yaw_4_6_pedal_yaw_4] -> Self_checking_pair_PedalYaw4/6(In6)
  pedal_yaw_4/6 -> [pedal_yaw_4_6_pedal_yaw_4]  "<pedal_yaw_4>"
  [pedal_yaw_4_7_pedal_yaw_4] -> Self_checking_pair_PedalYaw4/7(In7)
  pedal_yaw_4/7 -> [pedal_yaw_4_7_pedal_yaw_4]  "<pedal_yaw_4>"
  [pedal_yaw_4_8_pedal_yaw_4] -> Self_checking_pair_PedalYaw4/8(In8)
  pedal_yaw_4/8 -> [pedal_yaw_4_8_pedal_yaw_4]  "<pedal_yaw_4>"
  Self_checking_pair_SSC2_roll4/1(Out) -> [sidestick2Roll4_cmd]
  Self_checking_pair_SSC2_roll3/1(Out) -> [sidestick2Roll3_cmd]
  Self_checking_pair_SSC2_roll2/1(Out) -> [sidestick2Roll2_cmd]
  Self_checking_pair_SSC2_roll1/1(Out) -> [sidestick2Roll1_cmd]
  Self_checking_pair_SSC1_roll4/1(Out) -> [sidestick1Roll4_cmd]
  Self_checking_pair_SSC1_roll3/1(Out) -> [sidestick1Roll3_cmd]
  Self_checking_pair_SSC1_roll2/1(Out) -> [sidestick1Roll2_cmd]
  [sidestick1_roll_1_5_sidestick1_roll_1] -> Self_checking_pair_SSC1_roll1/5(In5)
  sidestick1_roll_1/5 -> [sidestick1_roll_1_5_sidestick1_roll_1]  "<sidestick1_roll_1>"
  [sidestick1_roll_1_6_sidestick1_roll_1] -> Self_checking_pair_SSC1_roll1/6(In6)
  sidestick1_roll_1/6 -> [sidestick1_roll_1_6_sidestick1_roll_1]  "<sidestick1_roll_1>"
  [sidestick1_roll_1_7_sidestick1_roll_1] -> Self_checking_pair_SSC1_roll1/7(In7)
  sidestick1_roll_1/7 -> [sidestick1_roll_1_7_sidestick1_roll_1]  "<sidestick1_roll_1>"
  [sidestick1_roll_1_8_sidestick1_roll_1] -> Self_checking_pair_SSC1_roll1/8(In8)
  sidestick1_roll_1/8 -> [sidestick1_roll_1_8_sidestick1_roll_1]  "<sidestick1_roll_1>"
  Self_checking_pair_SSC1_roll1/1(Out) -> [sidestick1Roll1_cmd]
  Self_checking_pair_SSC2_pitch4/1(Out) -> [sidestick2Pitch4_cmd]
  Self_checking_pair_SSC2_pitch3/1(Out) -> [sidestick2Pitch3_cmd]
  Self_checking_pair_SSC2_pitch2/1(Out) -> [sidestick2Pitch2_cmd]
  Self_checking_pair_SSC2_pitch1/1(Out) -> [sidestick2Pitch1_cmd]
  Self_checking_pair_SSC1_pitch4/1(Out) -> [sidestick1Pitch4_cmd]
  Self_checking_pair_SSC1_pitch3/1(Out) -> [sidestick1Pitch3_cmd]
  Self_checking_pair_SSC1_pitch2/1(Out) -> [sidestick1Pitch2_cmd]
  Self_checking_pair_SSC1_pitch1/1(Out) -> [sidestick1Pitch1_cmd]
  [sidestick1_pitch_1_1_sidestick1_pitch_1] -> Self_checking_pair_SSC1_pitch1/1(In1)
  sidestick1_pitch_1/1 -> [sidestick1_pitch_1_1_sidestick1_pitch_1]  "<sidestick1_pitch_1>"
  [sidestick1_pitch_1_2_sidestick1_pitch_1] -> Self_checking_pair_SSC1_pitch1/2(In2)
  sidestick1_pitch_1/2 -> [sidestick1_pitch_1_2_sidestick1_pitch_1]  "<sidestick1_pitch_1>"
  [sidestick1_pitch_1_3_sidestick1_pitch_1] -> Self_checking_pair_SSC1_pitch1/3(In3)
  sidestick1_pitch_1/3 -> [sidestick1_pitch_1_3_sidestick1_pitch_1]  "<sidestick1_pitch_1>"